simulink model "SdbRxSigProc"  (R2022b)
settings: Solver=FixedStepDiscrete  StartTime=0.0  StopTime=10.0  FixedStep=0.05  RelTol=1e-3
(part 3 of 4)

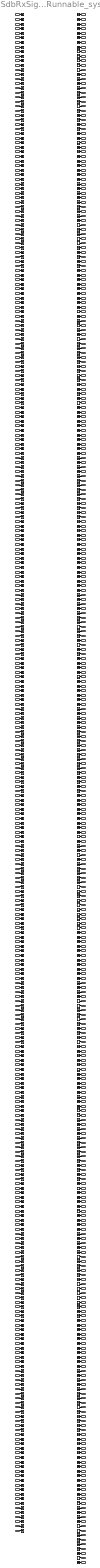
== system SdbRxSigProc/SdbRxSigProc_Runnable_sys ==
  ActvnOfWshrFrntSafe_ActvnOfWshrFrntSafe_read : Inport
  AirTFromHeatgEstimd_AirTFromHeatgEstimd_read : Inport  Port=2
  AmbTRaw_AmbTRaw_read : Inport  Port=3
  BltLockStAtDrvr_BltLockStAtDrvr_read : Inport  Port=4
  BltLockStAtPass_BltLockStAtPass_read : Inport  Port=5
  BltLockStAtRowSecLe_BltLockStAtRowSecLe_read : Inport  Port=6
  BltLockStAtRowSecMid_BltLockStAtRowSecMid_read : Inport  Port=7
  BltLockStAtRowSecRi_BltLockStAtRowSecRi_read : Inport  Port=8
  BltLockStAtRowThrdLe_BltLockStAtRowThrdLe_read : Inport  Port=9
  BltLockStAtRowThrdRi_BltLockStAtRowThrdRi_read : Inport  Port=10
  BookTrvlClimaActvReq_BookTrvlClimaActvReq_read : Inport  Port=11
  CamSts_CamSts_read : Inport  Port=12
  CarTiGlb_CarTiGlb_read : Inport  Port=13
  ClimaCfgForSnsrHumPrsnt1_ClimaCfgForSnsrHumPrsnt1_read : Inport  Port=14
  ClimaCmd_ClimaCmd_read : Inport  Port=15
  ClimaPwrAllwd_ClimaPwrAllwd_read : Inport  Port=16
  CmpmtInAirPmAQI_CmpmtInAirPmAQI_read : Inport  Port=17
  CmpmtInAirPmDnsty_CmpmtInAirPmDnsty_read : Inport  Port=18
  CmptmtCoolgSts_CmptmtCoolgSts_read : Inport  Port=19
  CmptmtFrntWindDewT_CmptmtFrntWindDewT_read : Inport  Port=20
  CmptmtFrntWindT_CmptmtFrntWindT_read : Inport  Port=21
  CmptmtRelHum_CmptmtRelHum_read : Inport  Port=22
  CoolgLoadGroup_CoolgLoadGroup_read : Inport  Port=23
  DefrstSts_DefrstSts_read : Inport  Port=24
  DoorDrvrSts_DoorDrvrSts_read : Inport  Port=25
  DoorLeReSts_DoorLeReSts_read : Inport  Port=26
  DoorPassSts_DoorPassSts_read : Inport  Port=27
  DoorRiReSts_DoorRiReSts_read : Inport  Port=28
  DrvModReq_DrvModReq_read : Inport  Port=29
  DrvrAirCdnrSetg_DrvrAirCdnrSetg_read : Inport  Port=30
  DrvrSeatHeatgActlPwrCns_DrvrSeatHeatgActlPwrCns_read : Inport  Port=31
  DrvrSeatHeatgDesPwrCns_DrvrSeatHeatgDesPwrCns_read : Inport  Port=32
  DrvrSeatVentnActlPwrCns_DrvrSeatVentnActlPwrCns_read : Inport  Port=33
  DrvrSeatVentnDesPwrCns_DrvrSeatVentnDesPwrCns_read : Inport  Port=34
  EgyCnsAllwdForClima_EgyCnsAllwdForClima_read : Inport  Port=35
  EngT_EngT_read : Inport  Port=36
  HMIClimaEgySaveReq_HMIClimaEgySaveReq_read : Inport  Port=37
  HpcDeiceReq_HpcDeiceReq_read : Inport  Port=38
  HvacFanSts2HvacFanBattI2_HvacFanSts2HvacFanBattI2_read : Inport  Port=39
  HvacFanSts2HvacFanMotU2_HvacFanSts2HvacFanMotU2_read : Inport  Port=40
  HvacFanSts3HvacFanBattI_HvacFanSts3HvacFanBattI_read : Inport  Port=41
  HvacFanSts3HvacFanSpdFd_HvacFanSts3HvacFanSpdFd_read : Inport  Port=42
  HvPwrEgyPrio_HvPwrEgyPrio_read : Inport  Port=43
  IHUAPSts_IHUAPSts_read : Inport  Port=44
  IncomingAirQlyCtrlFromHmi_IncomingAirQlyCtrlFromHmi_read : Inport  Port=45
  IncomingAirQlyPopUpReq_IncomingAirQlyPopUpReq_read : Inport  Port=46
  InPm25FanErr_InPm25FanErr_read : Inport  Port=47
  InPm25SnsrElecErr_InPm25SnsrElecErr_read : Inport  Port=48
  InPm25SnsrSts_InPm25SnsrSts_read : Inport  Port=49
  InteCleanUnpleSmell_InteCleanUnpleSmell_read : Inport  Port=50
  IsgMotT_IsgMotT_read : Inport  Port=51
  LeSolarData_LeSolarData_read : Inport  Port=52
  LockgCenSts_LockgCenSts_read : Inport  Port=53
  OnBdChrgrHndlSts1_OnBdChrgrHndlSts1_read : Inport  Port=54
  PassSeatHeatgActlPwrCns_PassSeatHeatgActlPwrCns_read : Inport  Port=55
  PassSeatHeatgDesPwrCns_PassSeatHeatgDesPwrCns_read : Inport  Port=56
  PassSeatVentnActlPwrCns_PassSeatVentnActlPwrCns_read : Inport  Port=57
  PassSeatVentnDesPwrCns_PassSeatVentnDesPwrCns_read : Inport  Port=58
  PostDrvgClimaReq_PostDrvgClimaReq_read : Inport  Port=59
  RainfallAmnt_RainfallAmnt_read : Inport  Port=60
  RelHumSnsrQf_RelHumSnsrQf_read : Inport  Port=61
  RemStrtHvCtrlReq_RemStrtHvCtrlReq_read : Inport  Port=62
  RiSolarData_RiSolarData_read : Inport  Port=63
  SceneModSeld_SceneModSeld_read : Inport  Port=64
  SeatHeatDurgClimaEnadFromTelm_SeatHeatDurgClimaEnadFromTelm_read : Inport  Port=65
  SecRowSeatHeatgActlPwrCnsLe_SecRowSeatHeatgActlPwrCnsLe_read : Inport  Port=66
  SecRowSeatHeatgActlPwrCnsRi_SecRowSeatHeatgActlPwrCnsRi_read : Inport  Port=67
  SecRowSeatHeatgDesPwrCnsLe_SecRowSeatHeatgDesPwrCnsLe_read : Inport  Port=68
  SecRowSeatHeatgDesPwrCnsRi_SecRowSeatHeatgDesPwrCnsRi_read : Inport  Port=69
  SecRowSeatVentnActlPwrCnsLe_SecRowSeatVentnActlPwrCnsLe_read : Inport  Port=70
  SecRowSeatVentnActlPwrCnsRi_SecRowSeatVentnActlPwrCnsRi_read : Inport  Port=71
  SecRowSeatVentnDesPwrCnsLe_SecRowSeatVentnDesPwrCnsLe_read : Inport  Port=72
  SecRowSeatVentnDesPwrCnsRi_SecRowSeatVentnDesPwrCnsRi_read : Inport  Port=73
  SolarSnsrVluQf_SolarSnsrVluQf_read : Inport  Port=74
  SteerWhlHeatgDesPwr_SteerWhlHeatgDesPwr_read : Inport  Port=75
  SteerWhlHeatgPwrAct_SteerWhlHeatgPwrAct_read : Inport  Port=76
  StrtRemSts1WdStrtRemSts_StrtRemSts1WdStrtRemSts_read : Inport  Port=77
  SunCurtPosnSts_SunCurtPosnSts_read : Inport  Port=78
  SunRoofPosnSts_SunRoofPosnSts_read : Inport  Port=79
  TelmPM25Req_TelmPM25Req_read : Inport  Port=80
  TrSts_TrSts_read : Inport  Port=81
  VehBattU_VehBattU_read : Inport  Port=82
  VehSpdLgt_VehSpdLgt_read : Inport  Port=83
  VehTiAndData_VehTiAndData_read : Inport  Port=84
  WadeProtnSts_WadeProtnSts_read : Inport  Port=85
  WinGlbCmd_WinGlbCmd_read : Inport  Port=86
  WinPosnStsAtDrvr_WinPosnStsAtDrvr_read : Inport  Port=87
  WinPosnStsAtPass_WinPosnStsAtPass_read : Inport  Port=88
  WinPosnStsAtReLe_WinPosnStsAtReLe_read : Inport  Port=89
  WinPosnStsAtReRi_WinPosnStsAtReRi_read : Inport  Port=90
  WipgInfo_WipgInfo_read : Inport  Port=91
  VehModMngtGlbSafe1_VehModMngtGlbSafe1_read : Inport  Port=92
  VehModMngtGlbSafe1_VehModMngtGlbSafe1_IsUpdated_read : Inport  Port=93
  AACAirClngErrSts_AACAirClngErrSts_read : Inport  Port=94
  AACAirClngSts_AACAirClngSts_read : Inport  Port=95
  AFUFragraCh1AvlTiPrec_AFUFragraCh1AvlTiPrec_read : Inport  Port=96
  AFUFragraCh1AvlTiPrec_AFUFragraCh1AvlTiPrec_IsUpdated_read : Inport  Port=97
  AFUFragraCh2AvlTiPrec_AFUFragraCh2AvlTiPrec_read : Inport  Port=98
  AFUFragraCh2AvlTiPrec_AFUFragraCh2AvlTiPrec_IsUpdated_read : Inport  Port=99
  AFUFragraCh3AvlTiPrec_AFUFragraCh3AvlTiPrec_read : Inport  Port=100
  AFUFragraCh3AvlTiPrec_AFUFragraCh3AvlTiPrec_IsUpdated_read : Inport  Port=101
  AFUFragraCh4AvlTiPrec_AFUFragraCh4AvlTiPrec_read : Inport  Port=102
  AFUFragraCh4AvlTiPrec_AFUFragraCh4AvlTiPrec_IsUpdated_read : Inport  Port=103
  AFUFragraCh5AvlTiPrec_AFUFragraCh5AvlTiPrec_read : Inport  Port=104
  AFUFragraCh5AvlTiPrec_AFUFragraCh5AvlTiPrec_IsUpdated_read : Inport  Port=105
  AirFragCh1OvrdDy_AirFragCh1OvrdDy_read : Inport  Port=106
  AirFragCh2OvrdDy_AirFragCh2OvrdDy_read : Inport  Port=107
  AirFragCh3OvrdDy_AirFragCh3OvrdDy_read : Inport  Port=108
  AirFragCh4OvrdDy_AirFragCh4OvrdDy_read : Inport  Port=109
  AirFragCh5OvrdDy_AirFragCh5OvrdDy_read : Inport  Port=110
  AirPtcEnad_AirPtcEnad_read : Inport  Port=111
  AirPtcHVPwrActl_AirPtcHVPwrActl_read : Inport  Port=112
  AirPtcHVPwrActlFristLe_AirPtcHVPwrActlFristLe_read : Inport  Port=113
  AirPtcHVPwrActlFristRi_AirPtcHVPwrActlFristRi_read : Inport  Port=114
  AirPtcHVPwrActlSecLe_AirPtcHVPwrActlSecLe_read : Inport  Port=115
  AirPtcModeReq_AirPtcModeReq_read : Inport  Port=116
  AirSrcStsFb_AirSrcStsFb_read : Inport  Port=117
  AltitudeInfrom_AltitudeInfrom_read : Inport  Port=118
  AMBSts_AMBSts_read : Inport  Port=119
  BackflushVlvErr_BackflushVlvErr_read : Inport  Port=120
  BusCurrentFb_BusCurrentFb_read : Inport  Port=121
  ChgFilElmWarn_ChgFilElmWarn_read : Inport  Port=122
  ClimaCfgForDrvrSeatVentWthSmf2_ClimaCfgForDrvrSeatVentWthSmf2_read : Inport  Port=123
  ClimaCfgForPassSeatVentWthSmf2_ClimaCfgForPassSeatVentWthSmf2_read : Inport  Port=124
  CmptmtOxygenConcQF_CmptmtOxygenConcQF_read : Inport  Port=125
  CmptmtOxygenConcVlu_CmptmtOxygenConcVlu_read : Inport  Port=126
  CoolgFanErr_CoolgFanErr_read : Inport  Port=127
  CourseDirFromNav_CourseDirFromNav_read : Inport  Port=128
  CurrentCongestionInformation_CurrentCongestionInformation_read : Inport  Port=129
  CurrentTunnelInformation_CurrentTunnelInformation_read : Inport  Port=130
  DcDcActvd_DcDcActvd_read : Inport  Port=131
  DcDcPwrLim_DcDcPwrLim_read : Inport  Port=132
  DHUResdSig10_DHUResdSig10_read : Inport  Port=133
  DHUResdSig11_DHUResdSig11_read : Inport  Port=134
  DHUResdSig12_DHUResdSig12_read : Inport  Port=135
  DHUResdSig13_DHUResdSig13_read : Inport  Port=136
  DHUResdSig3_DHUResdSig3_read : Inport  Port=137
  DHUResdSig4_DHUResdSig4_read : Inport  Port=138
  DHUResdSig5_DHUResdSig5_read : Inport  Port=139
  DHUResdSig6_DHUResdSig6_read : Inport  Port=140
  DHUResdSig7_DHUResdSig7_read : Inport  Port=141
  DHUResdSig8_DHUResdSig8_read : Inport  Port=142
  DHUResdSig9_DHUResdSig9_read : Inport  Port=143
  DispHvBattLvlOfChrg_DispHvBattLvlOfChrg_read : Inport  Port=144
  DropTopPosnSts_DropTopPosnSts_read : Inport  Port=145
  DrvModReqType1ForCompile_DrvModReqType1ForCompile_read : Inport  Port=146
  DrvrSeatPosPerc_DrvrSeatPosPerc_read : Inport  Port=147
  DrvrSeatPosPerc_DrvrSeatPosPerc_IsUpdated_read : Inport  Port=148
  DrvrSeatSts_DrvrSeatSts_read : Inport  Port=149
  DrvrSeatVentnActlPwrCns2_DrvrSeatVentnActlPwrCns2_read : Inport  Port=150
  DrvrSeatVentnDesPwrCns2_DrvrSeatVentnDesPwrCns2_read : Inport  Port=151
  DrvrSeatVentnLvlSts2_DrvrSeatVentnLvlSts2_read : Inport  Port=152
  EngSt1WdSts_EngSt1WdSts_read : Inport  Port=153
  EngSt1WdSts_EngSt1WdSts_IsUpdated_read : Inport  Port=154
  FctvSts_FctvSts_read : Inport  Port=155
  FloatDrvingActv_FloatDrvingActv_read : Inport  Port=156
  FrntAirPtcHVPwrActl_FrntAirPtcHVPwrActl_read : Inport  Port=157
  FrntAirPtcHVPwrActl_FrntAirPtcHVPwrActl_IsUpdated_read : Inport  Port=158
  HighTempCleanHmiReq_HighTempCleanHmiReq_read : Inport  Port=159
  HmiAC_HmiAC_read : Inport  Port=160
  HmiAC_HmiAC_IsUpdated_read : Inport  Port=161
  HMIAirCdnrAutDrySet_HMIAirCdnrAutDrySet_read : Inport  Port=162
  HMIAirCdnrAutDrySet_HMIAirCdnrAutDrySet_IsUpdated_read : Inport  Port=163
  HmiAirDistbnRow1_HmiAirDistbnRow1_read : Inport  Port=164
  HmiAirDistbnRow1_HmiAirDistbnRow1_IsUpdated_read : Inport  Port=165
  HmiAirDistbnRow1Le_HmiAirDistbnRow1Le_read : Inport  Port=166
  HmiAirDistbnRow1Ri_HmiAirDistbnRow1Ri_read : Inport  Port=167
  HmiAirDistbnRow2_HmiAirDistbnRow2_read : Inport  Port=168
  HmiAirDistbnRow2_HmiAirDistbnRow2_IsUpdated_read : Inport  Port=169
  HmiAutoRow1_HmiAutoRow1_read : Inport  Port=170
  HmiAutoRow1_HmiAutoRow1_IsUpdated_read : Inport  Port=171
  HmiAutoRow1Le_HmiAutoRow1Le_read : Inport  Port=172
  HmiAutoRow1Ri_HmiAutoRow1Ri_read : Inport  Port=173
  HmiAutoRow2_HmiAutoRow2_read : Inport  Port=174
  HmiAutoRow2_HmiAutoRow2_IsUpdated_read : Inport  Port=175
  HmiAutOxySet_HmiAutOxySet_read : Inport  Port=176
  HMIBluetoothCallToAdjAirVol_HMIBluetoothCallToAdjAirVol_read : Inport  Port=177
  HmiCarLockACRecircSet_HmiCarLockACRecircSet_read : Inport  Port=178
  HmiCbnOverHeatPrevnReq_HmiCbnOverHeatPrevnReq_read : Inport  Port=179
  HmiCbnOverHeatPrevnReq_HmiCbnOverHeatPrevnReq_IsUpdated_read : Inport  Port=180
  HmiClimaRow1LePwr_HmiClimaRow1LePwr_read : Inport  Port=181
  HmiClimaRow1Pwr_HmiClimaRow1Pwr_read : Inport  Port=182
  HmiClimaRow1RiPwr_HmiClimaRow1RiPwr_read : Inport  Port=183
  HmiClimaRow2Pwr_HmiClimaRow2Pwr_read : Inport  Port=184
  HmiClimaRow3Pwr_HmiClimaRow3Pwr_read : Inport  Port=185
  HMIClimaVentSet_HMIClimaVentSet_read : Inport  Port=186
  HmiCmptmtAirDistbnFrnt_HmiCmptmtAirDistbnFrnt_read : Inport  Port=187
  HmiDrvrHeadPosn_HmiDrvrHeadPosn_read : Inport  Port=188
  HmiEcoClimaReq_HmiEcoClimaReq_read : Inport  Port=189
  HmiEcoClimaReq_HmiEcoClimaReq_IsUpdated_read : Inport  Port=190
  HmiElecAirBodyVentLebtn_HmiElecAirBodyVentLebtn_read : Inport  Port=191
  HmiElecAirBodyVentRibtn_HmiElecAirBodyVentRibtn_read : Inport  Port=192
  HmiElecAirConsolePosn_HmiElecAirConsolePosn_read : Inport  Port=193
  HmiElecAirConsoleSwtLe_HmiElecAirConsoleSwtLe_read : Inport  Port=194
  HmiElecAirConsoleSwtRi_HmiElecAirConsoleSwtRi_read : Inport  Port=195
  HmiElecAirDirModeSecRowReq_HmiElecAirDirModeSecRowReq_read : Inport  Port=196
  HmiElecAirDirSwngSecLe_HmiElecAirDirSwngSecLe_read : Inport  Port=197
  HmiElecAirDirSwngSecRi_HmiElecAirDirSwngSecRi_read : Inport  Port=198
  HmiElecAirDrvrNatureWindReq_HmiElecAirDrvrNatureWindReq_read : Inport  Port=199
  HmiElecAirPassNatureWindReq_HmiElecAirPassNatureWindReq_read : Inport  Port=200
  HMIFACSwt_HMIFACSwt_read : Inport  Port=201
  HmiFanLvlRow1_HmiFanLvlRow1_read : Inport  Port=202
  HmiFanLvlRow1_HmiFanLvlRow1_IsUpdated_read : Inport  Port=203
  HmiFanLvlRow1Le_HmiFanLvlRow1Le_read : Inport  Port=204
  HmiFanLvlRow1Ri_HmiFanLvlRow1Ri_read : Inport  Port=205
  HmiFanLvlRow2_HmiFanLvlRow2_read : Inport  Port=206
  HmiFanLvlRow2_HmiFanLvlRow2_IsUpdated_read : Inport  Port=207
  HmiFragraCh_HmiFragraCh_read : Inport  Port=208
  HmiFragraCh_HmiFragraCh_IsUpdated_read : Inport  Port=209
  HmiFragraLvl_HmiFragraLvl_read : Inport  Port=210
  HmiFragraLvl_HmiFragraLvl_IsUpdated_read : Inport  Port=211
  HMIIonizerAirCdnr_HMIIonizerAirCdnr_read : Inport  Port=212
  HMIIonizerAirCdnr_HMIIonizerAirCdnr_IsUpdated_read : Inport  Port=213
  HMILBRACStrtSet_HMILBRACStrtSet_read : Inport  Port=214
  HmiMaxAC_HmiMaxAC_read : Inport  Port=215
  HmiMaxAC_HmiMaxAC_IsUpdated_read : Inport  Port=216
  HmiMaxDefrst_HmiMaxDefrst_read : Inport  Port=217
  HmiMaxDefrst_HmiMaxDefrst_IsUpdated_read : Inport  Port=218
  HmiOxyinCarSwt_HmiOxyinCarSwt_read : Inport  Port=219
  HmiParkVentSet_HmiParkVentSet_read : Inport  Port=220
  HmiParkVentTmrSet_HmiParkVentTmrSet_read : Inport  Port=221
  HmiPassHeadPosn_HmiPassHeadPosn_read : Inport  Port=222
  HmiPM25PopUpResp_HmiPM25PopUpResp_read : Inport  Port=223
  HmiPM25PopUpResp_HmiPM25PopUpResp_IsUpdated_read : Inport  Port=224
  HmiPM25PopUpSet_HmiPM25PopUpSet_read : Inport  Port=225
  HmiPM25PopUpSet_HmiPM25PopUpSet_IsUpdated_read : Inport  Port=226
  HmiSmrtWindFolwSet_HmiSmrtWindFolwSet_read : Inport  Port=227
  HMIThermoTankDoorOpen_HMIThermoTankDoorOpen_read : Inport  Port=228
  HMIThermoTankEcoSw_HMIThermoTankEcoSw_read : Inport  Port=229
  HMIThermoTankT_HMIThermoTankT_read : Inport  Port=230
  HmiThermoTSteDeoNewSwt_HmiThermoTSteDeoNewSwt_read : Inport  Port=231
  HmiThermoTSteDeoSwt_HmiThermoTSteDeoSwt_read : Inport  Port=232
  HmiTSyncn_HmiTSyncn_read : Inport  Port=233
  HmiTSyncn_HmiTSyncn_IsUpdated_read : Inport  Port=234
  HmiUVSterilSet_HmiUVSterilSet_read : Inport  Port=235
  HmiWinterLockCarPrevnIceSet_HmiWinterLockCarPrevnIceSet_read : Inport  Port=236
  HmiWinterLockCarPrevnIceSet_HmiWinterLockCarPrevnIceSet_IsUpdated_read : Inport  Port=237
  HoodSts_HoodSts_read : Inport  Port=238
  HvBattLimnIndcn_HvBattLimnIndcn_read : Inport  Port=239
  InflateAirSrcSwt_InflateAirSrcSwt_read : Inport  Port=240
  InpVoltFb_InpVoltFb_read : Inport  Port=241
  LiOprnMod_LiOprnMod_read : Inport  Port=242
  LonglifeBatteryReq_LonglifeBatteryReq_read : Inport  Port=243
  McuOvrTempErr_McuOvrTempErr_read : Inport  Port=244
  McuTempFb_McuTempFb_read : Inport  Port=245
  McuTempSnsrErr_McuTempSnsrErr_read : Inport  Port=246
  MoleculeVlv1_MoleculeVlv1_read : Inport  Port=247
  MoleculeVlv2_MoleculeVlv2_read : Inport  Port=248
  MosOvrTempErr_MosOvrTempErr_read : Inport  Port=249
  MosTempFb_MosTempFb_read : Inport  Port=250
  MosTempSnsrErr_MosTempSnsrErr_read : Inport  Port=251
  MotStsFrmOGM_MotStsFrmOGM_read : Inport  Port=252
  MotTempFb_MotTempFb_read : Inport  Port=253
  MotTempSnsrErr_MotTempSnsrErr_read : Inport  Port=254
  OGMCmprSpdFb_OGMCmprSpdFb_read : Inport  Port=255
  OGMFlowFb_OGMFlowFb_read : Inport  Port=256
  OGMHwVers_OGMHwVers_read : Inport  Port=257
  OGMIntErr_OGMIntErr_read : Inport  Port=258
  OGMOvrVoltRangErr_OGMOvrVoltRangErr_read : Inport  Port=259
  OGMRunTi_OGMRunTi_read : Inport  Port=260
  OGMSwVers_OGMSwVers_read : Inport  Port=261
  OGMTempErr_OGMTempErr_read : Inport  Port=262
  OGMUndVoltRangErr_OGMUndVoltRangErr_read : Inport  Port=263
  OxygenConcFbFrmOGM_OxygenConcFbFrmOGM_read : Inport  Port=264
  OxygenConcFlt_OxygenConcFlt_read : Inport  Port=265
  OxygenConcSnsrElecErr_OxygenConcSnsrElecErr_read : Inport  Port=266
  OxygenConcSnsrIntErr_OxygenConcSnsrIntErr_read : Inport  Port=267
  OxygenConcSnsrOvrVoltRangErr_OxygenConcSnsrOvrVoltRangErr_read : Inport  Port=268
  OxygenConcSnsrSts_OxygenConcSnsrSts_read : Inport  Port=269
  OxygenConcSnsrStsFrmOGM_OxygenConcSnsrStsFrmOGM_read : Inport  Port=270
  OxygenConcSnsrTempErr_OxygenConcSnsrTempErr_read : Inport  Port=271
  OxygenConcSnsrUndVoltRangErr_OxygenConcSnsrUndVoltRangErr_read : Inport  Port=272
  OxygenGenrErrInfo_OxygenGenrErrInfo_read : Inport  Port=273
  OxygenSplyAvlSts_OxygenSplyAvlSts_read : Inport  Port=274
  OxygenSplyErr_OxygenSplyErr_read : Inport  Port=275
  OxygenTubeBlk_OxygenTubeBlk_read : Inport  Port=276
  PassSeatPosPerc_PassSeatPosPerc_read : Inport  Port=277
  PassSeatPosPerc_PassSeatPosPerc_IsUpdated_read : Inport  Port=278
  PassSeatSts2_PassSeatSts2_read : Inport  Port=279
  PassSeatVentnActlPwrCns2_PassSeatVentnActlPwrCns2_read : Inport  Port=280
  PassSeatVentnDesPwrCns2_PassSeatVentnDesPwrCns2_read : Inport  Port=281
  PassSeatVentnLvlSts2_PassSeatVentnLvlSts2_read : Inport  Port=282
  PhaseCurrentFb_PhaseCurrentFb_read : Inport  Port=283
  PosnBrief_PosnBrief_read : Inport  Port=284
  PressureFb_PressureFb_read : Inport  Port=285
  PressureFlt_PressureFlt_read : Inport  Port=286
  PressureSnsrErr_PressureSnsrErr_read : Inport  Port=287
  RefrLpSwSts_RefrLpSwSts_read : Inport  Port=288
  RefrtorVlvEna_RefrtorVlvEna_read : Inport  Port=289
  ResrvdSigForCCM2_ResrvdSigForCCM2_read : Inport  Port=290
  RoadIncln_RoadIncln_read : Inport  Port=291
  RuranACModeSet_RuranACModeSet_read : Inport  Port=292
  SeatBackAngleRowFirstDrvr_SeatBackAngleRowFirstDrvr_read : Inport  Port=293
  SeatBackAngleRowFirstDrvr_SeatBackAngleRowFirstDrvr_IsUpdated_read : Inport  Port=294
  SeatBackAngleRowFirstPass_SeatBackAngleRowFirstPass_read : Inport  Port=295
  SeatBackAngleRowFirstPass_SeatBackAngleRowFirstPass_IsUpdated_read : Inport  Port=296
  SeatOccptAtRowSecDrSide2_SeatOccptAtRowSecDrSide2_read : Inport  Port=297
  SeatOccptAtRowSecMid2_SeatOccptAtRowSecMid2_read : Inport  Port=298
  SeatOccptAtRowSecPasSide2_SeatOccptAtRowSecPasSide2_read : Inport  Port=299
  SeatOccptAtRowThrdLe2_SeatOccptAtRowThrdLe2_read : Inport  Port=300
  SeatOccptAtRowThrdRi2_SeatOccptAtRowThrdRi2_read : Inport  Port=301
  SportModeThmgtRequest_SportModeThmgtRequest_read : Inport  Port=302
  TCSCooltFlow_TCSCooltFlow_read : Inport  Port=303
  TCSCooltT_TCSCooltT_read : Inport  Port=304
  TcsDoorEleBailReq_TcsDoorEleBailReq_read : Inport  Port=305
  TcsFanReq_TcsFanReq_read : Inport  Port=306
  TcsModSwtReq_TcsModSwtReq_read : Inport  Port=307
  TcsOvrheatdProtnSts_TcsOvrheatdProtnSts_read : Inport  Port=308
  TcsSemiconReq_TcsSemiconReq_read : Inport  Port=309
  TcsTmrOverReq_TcsTmrOverReq_read : Inport  Port=310
  TcsTNowCtrlReq_TcsTNowCtrlReq_read : Inport  Port=311
  TcsTopDoorEleBailReq_TcsTopDoorEleBailReq_read : Inport  Port=312
  TelmOxygenValReq_TelmOxygenValReq_read : Inport  Port=313
  TelmThermoTankECOSet_TelmThermoTankECOSet_read : Inport  Port=314
  TelmThermoTankT_TelmThermoTankT_read : Inport  Port=315
  ThermHvacRecircReq_ThermHvacRecircReq_read : Inport  Port=316
  ThermHvacRecircReqEna_ThermHvacRecircReqEna_read : Inport  Port=317
  ThrdRowSeatHeatgActlPwrCnsLe_ThrdRowSeatHeatgActlPwrCnsLe_read : Inport  Port=318
  ThrdRowSeatHeatgActlPwrCnsRi_ThrdRowSeatHeatgActlPwrCnsRi_read : Inport  Port=319
  ThrdRowSeatHeatgDesPwrCnsLe_ThrdRowSeatHeatgDesPwrCnsLe_read : Inport  Port=320
  ThrdRowSeatHeatgDesPwrCnsRi_ThrdRowSeatHeatgDesPwrCnsRi_read : Inport  Port=321
  ThrdRowSeatVentnActlPwrCnsLe_ThrdRowSeatVentnActlPwrCnsLe_read : Inport  Port=322
  ThrdRowSeatVentnActlPwrCnsRi_ThrdRowSeatVentnActlPwrCnsRi_read : Inport  Port=323
  ThrdRowSeatVentnDesPwrCnsLe_ThrdRowSeatVentnDesPwrCnsLe_read : Inport  Port=324
  ThrdRowSeatVentnDesPwrCnsRi_ThrdRowSeatVentnDesPwrCnsRi_read : Inport  Port=325
  UseEvapReqTMinNew_UseEvapReqTMinNew_read : Inport  Port=326
  VlvVoltFlt_VlvVoltFlt_read : Inport  Port=327
  WarnLvlMsg_WarnLvlMsg_read : Inport  Port=328
  WarnLvlMsg_WarnLvlMsg_IsUpdated_read : Inport  Port=329
  WasRespLevel_WasRespLevel_read : Inport  Port=330
  WasRespLevel_WasRespLevel_IsUpdated_read : Inport  Port=331
  WaterDepthInformation_WaterDepthInformation_read : Inport  Port=332
  WeatherQualityAQI_WeatherQualityAQI_read : Inport  Port=333
  function : TriggerPort  TriggerType=function-call  VariantControl=(inherit)  SampleTimeType=periodic  SampleTime=-1
  Ground_{1..8,14..20,22..28,30..46,48..49,52..65,67..68,70..71,73..79,81..85,87..134,136..138,140..145,147..180,182..184,186..191,194,202..216,219..220,222..225,227..231,233..240,243..257,259..262,264..272,274..278,284..305,307..325,328..331,334..338,340} (290 alike) : Ground
  Ground_10 : Subsystem
  Ground_11 : Subsystem
  Ground_12 : Subsystem
  Ground_13 : Subsystem
  Ground_135 : Subsystem
  Ground_139 : Subsystem
  Ground_146 : Subsystem
  Ground_181 : Subsystem
  Ground_185 : Subsystem
  Ground_192 : Subsystem
  Ground_193 : Subsystem
  Ground_195 : Subsystem
  Ground_196 : Subsystem
  Ground_197 : Subsystem
  Ground_198 : Subsystem
  Ground_199 : Subsystem
  Ground_200 : Subsystem
  Ground_201 : Subsystem
  Ground_21 : Subsystem
  Ground_217 : Subsystem
  Ground_218 : Subsystem
  Ground_221 : Subsystem
  Ground_226 : Subsystem
  Ground_232 : Subsystem
  Ground_241 : Subsystem
  Ground_242 : Subsystem
  Ground_258 : Subsystem
  Ground_263 : Subsystem
  Ground_273 : Subsystem
  Ground_279 : Subsystem
  Ground_280 : Subsystem
  Ground_281 : Subsystem
  Ground_282 : Subsystem
  Ground_283 : Subsystem
  Ground_29 : Subsystem
  Ground_306 : Subsystem
  Ground_326 : Subsystem
  Ground_327 : Subsystem
  Ground_332 : Subsystem
  Ground_333 : Subsystem
  Ground_339 : Subsystem
  Ground_47 : Subsystem
  Ground_50 : Subsystem
  Ground_51 : Subsystem
  Ground_66 : Subsystem
  Ground_69 : Subsystem
  Ground_72 : Subsystem
  Ground_80 : Subsystem
  Ground_86 : Subsystem
  Ground_9 : Subsystem
  Terminator_{1..333} (333 alike) : Terminator
  AACAirClngErrStsNgcc_AACAirClngErrStsNgcc_write : Outport
      settings: EnsureOutportIsVirtual=on
  AACAirClngStsNgcc_AACAirClngStsNgcc_write : Outport  Port=2
      settings: EnsureOutportIsVirtual=on
  AFUFragraCh1AvlTiPrecNgcc_AFUFragraCh1AvlTiPrecNgcc_write : Outport  Port=3
      settings: EnsureOutportIsVirtual=on
  AFUFragraCh2AvlTiPrecNgcc_AFUFragraCh2AvlTiPrecNgcc_write : Outport  Port=4
      settings: EnsureOutportIsVirtual=on
  AFUFragraCh3AvlTiPrecNgcc_AFUFragraCh3AvlTiPrecNgcc_write : Outport  Port=5
      settings: EnsureOutportIsVirtual=on
  AFUFragraCh4AvlTiPrecNgcc_AFUFragraCh4AvlTiPrecNgcc_write : Outport  Port=6
      settings: EnsureOutportIsVirtual=on
  AFUFragraCh5AvlTiPrecNgcc_AFUFragraCh5AvlTiPrecNgcc_write : Outport  Port=7
      settings: EnsureOutportIsVirtual=on
  AirClngReq_AirClngReq_write : Outport  Port=8
      settings: EnsureOutportIsVirtual=on
  AirFragCh1OvrdDyNgcc_AirFragCh1OvrdDyNgcc_write : Outport  Port=9
      settings: EnsureOutportIsVirtual=on
  AirFragCh2OvrdDyNgcc_AirFragCh2OvrdDyNgcc_write : Outport  Port=10
      settings: EnsureOutportIsVirtual=on
  AirFragCh3OvrdDyNgcc_AirFragCh3OvrdDyNgcc_write : Outport  Port=11
      settings: EnsureOutportIsVirtual=on
  AirFragCh4OvrdDyNgcc_AirFragCh4OvrdDyNgcc_write : Outport  Port=12
      settings: EnsureOutportIsVirtual=on
  AirFragCh5OvrdDyNgcc_AirFragCh5OvrdDyNgcc_write : Outport  Port=13
      settings: EnsureOutportIsVirtual=on
  AirPtcEnadNgcc_AirPtcEnadNgcc_write : Outport  Port=14
      settings: EnsureOutportIsVirtual=on
  AirPtcHVPwrActlFirstLeNgcc_AirPtcHVPwrActlFirstLeNgcc_write : Outport  Port=15
      settings: EnsureOutportIsVirtual=on
  AirPtcHVPwrActlFirstRiNgcc_AirPtcHVPwrActlFirstRiNgcc_write : Outport  Port=16
      settings: EnsureOutportIsVirtual=on
  AirPtcHVPwrActlSecLeNgcc_AirPtcHVPwrActlSecLeNgcc_write : Outport  Port=17
      settings: EnsureOutportIsVirtual=on
  AirPtcModeReqNgcc_AirPtcModeReqNgcc_write : Outport  Port=18
      settings: EnsureOutportIsVirtual=on
  AirSrcStsFbNgcc_AirSrcStsFbNgcc_write : Outport  Port=19
      settings: EnsureOutportIsVirtual=on
  AltitudeInfromNgcc_AltitudeInfromNgcc_write : Outport  Port=20
      settings: EnsureOutportIsVirtual=on
  AMBStsNgcc_AMBStsNgcc_write : Outport  Port=21
      settings: EnsureOutportIsVirtual=on
  BackflushVlvErrNgcc_BackflushVlvErrNgcc_write : Outport  Port=22
      settings: EnsureOutportIsVirtual=on
  BookTrvlClimaActvReq_Ngcc_BookTrvlClimaActvReq_write : Outport  Port=23
      settings: EnsureOutportIsVirtual=on
  BusCurrentFbNgcc_BusCurrentFbNgcc_write : Outport  Port=24
      settings: EnsureOutportIsVirtual=on
  ChgFilElmWarnNgcc_ChgFilElmWarnNgcc_write : Outport  Port=25
      settings: EnsureOutportIsVirtual=on
  CmptmtOxygenConcQFNgcc_CmptmtOxygenConcQFNgcc_write : Outport  Port=26
      settings: EnsureOutportIsVirtual=on
  CmptmtOxygenConcVluNgcc_CmptmtOxygenConcVluNgcc_write : Outport  Port=27
      settings: EnsureOutportIsVirtual=on
  CoolgFanErrNgcc_CoolgFanErrNgcc_write : Outport  Port=28
      settings: EnsureOutportIsVirtual=on
  CourseDirFromNavNgcc_CourseDirFromNavNgcc_write : Outport  Port=29
      settings: EnsureOutportIsVirtual=on
  CurrentCongestionInformationNgcc_CurrentCongestionInformationNgcc_write : Outport  Port=30
      settings: EnsureOutportIsVirtual=on
  CurrentTunnelInformationNgcc_CurrentTunnelInformationNgcc_write : Outport  Port=31
      settings: EnsureOutportIsVirtual=on
  DcDcActvdNgcc_DcDcActvdNgcc_write : Outport  Port=32
      settings: EnsureOutportIsVirtual=on
  DcDcPwrLimNgcc_DcDcPwrLimNgcc_write : Outport  Port=33
      settings: EnsureOutportIsVirtual=on
  DHUResdSig10Ngcc_DHUResdSig10Ngcc_write : Outport  Port=34
      settings: EnsureOutportIsVirtual=on
  DHUResdSig11Ngcc_DHUResdSig11Ngcc_write : Outport  Port=35
      settings: EnsureOutportIsVirtual=on
  DHUResdSig12Ngcc_DHUResdSig12Ngcc_write : Outport  Port=36
      settings: EnsureOutportIsVirtual=on
  DHUResdSig13Ngcc_DHUResdSig13Ngcc_write : Outport  Port=37
      settings: EnsureOutportIsVirtual=on
  DHUResdSig3Ngcc_DHUResdSig3Ngcc_write : Outport  Port=38
      settings: EnsureOutportIsVirtual=on
  DHUResdSig4Ngcc_DHUResdSig4Ngcc_write : Outport  Port=39
      settings: EnsureOutportIsVirtual=on
  DHUResdSig5Ngcc_DHUResdSig5Ngcc_write : Outport  Port=40
      settings: EnsureOutportIsVirtual=on
  DHUResdSig6Ngcc_DHUResdSig6Ngcc_write : Outport  Port=41
      settings: EnsureOutportIsVirtual=on
  DHUResdSig7Ngcc_DHUResdSig7Ngcc_write : Outport  Port=42
      settings: EnsureOutportIsVirtual=on
  DHUResdSig8Ngcc_DHUResdSig8Ngcc_write : Outport  Port=43
      settings: EnsureOutportIsVirtual=on
  DHUResdSig9Ngcc_DHUResdSig9Ngcc_write : Outport  Port=44
      settings: EnsureOutportIsVirtual=on
  DispHvBattLvlOfChrgNgcc_DispHvBattLvlOfChrgNgcc_write : Outport  Port=45
      settings: EnsureOutportIsVirtual=on
  DropTopPosnStsNgcc_DropTopPosnStsNgcc_write : Outport  Port=46
      settings: EnsureOutportIsVirtual=on
  DrvrSeatPosPercNgcc_DrvrSeatPosPercNgcc_write : Outport  Port=47
      settings: EnsureOutportIsVirtual=on
  DrvrSeatSts_Ngcc_DrvrSeatSts_write : Outport  Port=48
      settings: EnsureOutportIsVirtual=on
  DrvrSeatVentnLvlSts2Ngcc_DrvrSeatVentnLvlSts2Ngcc_write : Outport  Port=49
      settings: EnsureOutportIsVirtual=on
  FctvStsNgcc_FctvStsNgcc_write : Outport  Port=50
      settings: EnsureOutportIsVirtual=on
  FloatDrvingActvNgcc_FloatDrvingActvNgcc_write : Outport  Port=51
      settings: EnsureOutportIsVirtual=on
  HighTempCleanHmiReqNgcc_HighTempCleanHmiReqNgcc_write : Outport  Port=52
      settings: EnsureOutportIsVirtual=on
  HMIAirCdnrAutDrySetNgcc_HMIAirCdnrAutDrySetNgcc_write : Outport  Port=53
      settings: EnsureOutportIsVirtual=on
  HmiAirDistbnRow1LeNgcc_HmiAirDistbnRow1LeNgcc_write : Outport  Port=54
      settings: EnsureOutportIsVirtual=on
  HmiAirDistbnRow1RiNgcc_HmiAirDistbnRow1RiNgcc_write : Outport  Port=55
      settings: EnsureOutportIsVirtual=on
  HmiAutoRow1LeNgcc_HmiAutoRow1LeNgcc_write : Outport  Port=56
      settings: EnsureOutportIsVirtual=on
  HmiAutoRow1RiNgcc_HmiAutoRow1RiNgcc_write : Outport  Port=57
      settings: EnsureOutportIsVirtual=on
  HmiAutOxySetNgcc_HmiAutOxySetNgcc_write : Outport  Port=58
      settings: EnsureOutportIsVirtual=on
  HmiCarLockACRecircSetNgcc_HmiCarLockACRecircSetNgcc_write : Outport  Port=59
      settings: EnsureOutportIsVirtual=on
  HmiClimaRow1LePwrNgcc_HmiClimaRow1LePwrNgcc_write : Outport  Port=60
      settings: EnsureOutportIsVirtual=on
  HmiClimaRow1PwrNgcc_HmiClimaRow1PwrNgcc_write : Outport  Port=61
      settings: EnsureOutportIsVirtual=on
  HmiClimaRow1RiPwrNgcc_HmiClimaRow1RiPwrNgcc_write : Outport  Port=62
      settings: EnsureOutportIsVirtual=on
  HmiClimaRow2PwrNgcc_HmiClimaRow2PwrNgcc_write : Outport  Port=63
      settings: EnsureOutportIsVirtual=on
  HmiClimaRow3PwrNgcc_HmiClimaRow3PwrNgcc_write : Outport  Port=64
      settings: EnsureOutportIsVirtual=on
  HMIClimaVentSetNgcc_HMIClimaVentSetNgcc_write : Outport  Port=65
      settings: EnsureOutportIsVirtual=on
  HmiDrvrHeadPosnNgcc_HmiDrvrHeadPosnNgcc_write : Outport  Port=66
      settings: EnsureOutportIsVirtual=on
  HmiElecAirBodyVentLebtnNgcc_HmiElecAirBodyVentLebtnNgcc_write : Outport  Port=67
      settings: EnsureOutportIsVirtual=on
  HmiElecAirBodyVentRibtnNgcc_HmiElecAirBodyVentRibtnNgcc_write : Outport  Port=68
      settings: EnsureOutportIsVirtual=on
  HmiElecAirConsolePosnNgcc_HmiElecAirConsolePosnNgcc_write : Outport  Port=69
      settings: EnsureOutportIsVirtual=on
  HmiElecAirConsoleSwtLeNgcc_HmiElecAirConsoleSwtLeNgcc_write : Outport  Port=70
      settings: EnsureOutportIsVirtual=on
  HmiElecAirConsoleSwtRiNgcc_HmiElecAirConsoleSwtRiNgcc_write : Outport  Port=71
      settings: EnsureOutportIsVirtual=on
  HmiElecAirDirModeSecRowReqNgcc_HmiElecAirDirModeSecRowReqNgcc_write : Outport  Port=72
      settings: EnsureOutportIsVirtual=on
  HmiElecAirDirSwngSecLeNgcc_HmiElecAirDirSwngSecLeNgcc_write : Outport  Port=73
      settings: EnsureOutportIsVirtual=on
  HmiElecAirDirSwngSecRiNgcc_HmiElecAirDirSwngSecRiNgcc_write : Outport  Port=74
      settings: EnsureOutportIsVirtual=on
  HmiElecAirDrvrNatureWindReqNgcc_HmiElecAirDrvrNatureWindReqNgcc_write : Outport  Port=75
      settings: EnsureOutportIsVirtual=on
  HmiElecAirPassNatureWindReqNgcc_HmiElecAirPassNatureWindReqNgcc_write : Outport  Port=76
      settings: EnsureOutportIsVirtual=on
  HMIFACSwtNgcc_HMIFACSwtNgcc_write : Outport  Port=77
      settings: EnsureOutportIsVirtual=on
  HmiFanLvlRow1LeNgcc_HmiFanLvlRow1LeNgcc_write : Outport  Port=78
      settings: EnsureOutportIsVirtual=on
  HmiFanLvlRow1RiNgcc_HmiFanLvlRow1RiNgcc_write : Outport  Port=79
      settings: EnsureOutportIsVirtual=on
  HmiFragraChRatReq_HmiFragraChRatReq_write : Outport  Port=80
      settings: EnsureOutportIsVirtual=on
  HmiFragraLvlReq_HmiFragraLvlReq_write : Outport  Port=81
      settings: EnsureOutportIsVirtual=on
  HMILBRACStrtSetNgcc_HMILBRACStrtSetNgcc_write : Outport  Port=82
      settings: EnsureOutportIsVirtual=on
  HmiOxyinCarSwtNgcc_HmiOxyinCarSwtNgcc_write : Outport  Port=83
      settings: EnsureOutportIsVirtual=on
  HmiParkVentSetNgcc_HmiParkVentSetNgcc_write : Outport  Port=84
      settings: EnsureOutportIsVirtual=on
  HmiParkVentTmrSetNgcc_HmiParkVentTmrSetNgcc_write : Outport  Port=85
      settings: EnsureOutportIsVirtual=on
  HmiPassHeadPosnNgcc_HmiPassHeadPosnNgcc_write : Outport  Port=86
      settings: EnsureOutportIsVirtual=on
  HmiPopUpResp_HmiPopUpResp_write : Outport  Port=87
      settings: EnsureOutportIsVirtual=on
  HmiSmrtWindFolwSetNgcc_HmiSmrtWindFolwSetNgcc_write : Outport  Port=88
      settings: EnsureOutportIsVirtual=on
  HMIThermoTankDoorOpenNgcc_HMIThermoTankDoorOpenNgcc_write : Outport  Port=89
      settings: EnsureOutportIsVirtual=on
  HMIThermoTankEcoSwNgcc_HMIThermoTankEcoSwNgcc_write : Outport  Port=90
      settings: EnsureOutportIsVirtual=on
  HMIThermoTankTNgcc_HMIThermoTankTNgcc_write : Outport  Port=91
      settings: EnsureOutportIsVirtual=on
  HmiThermoTSteDeoNewSwtNgcc_HmiThermoTSteDeoNewSwtNgcc_write : Outport  Port=92
      settings: EnsureOutportIsVirtual=on
  HmiThermoTSteDeoSwtNgcc_HmiThermoTSteDeoSwtNgcc_write : Outport  Port=93
      settings: EnsureOutportIsVirtual=on
  HmiUVSterilSetNgcc_HmiUVSterilSetNgcc_write : Outport  Port=94
      settings: EnsureOutportIsVirtual=on
  HmiWinterLockCarPrevnIceSetNgcc_HmiWinterLockCarPrevnIceSetNgcc_write : Outport  Port=95
      settings: EnsureOutportIsVirtual=on
  HoodStsNgcc_HoodStsNgcc_write : Outport  Port=96
      settings: EnsureOutportIsVirtual=on
  HvBattLimnIndcnNgcc_HvBattLimnIndcnNgcc_write : Outport  Port=97
      settings: EnsureOutportIsVirtual=on
  InflateAirSrcSwtNgcc_InflateAirSrcSwtNgcc_write : Outport  Port=98
      settings: EnsureOutportIsVirtual=on
  InpVoltFbNgcc_InpVoltFbNgcc_write : Outport  Port=99
      settings: EnsureOutportIsVirtual=on
  LiOprnModNgcc_LiOprnModNgcc_write : Outport  Port=100
      settings: EnsureOutportIsVirtual=on
  LonglifeBatteryReqNgcc_LonglifeBatteryReqNgcc_write : Outport  Port=101
      settings: EnsureOutportIsVirtual=on
  McuOvrTempErrNgcc_McuOvrTempErrNgcc_write : Outport  Port=102
      settings: EnsureOutportIsVirtual=on
  McuTempFbNgcc_McuTempFbNgcc_write : Outport  Port=103
      settings: EnsureOutportIsVirtual=on
  McuTempSnsrErrNgcc_McuTempSnsrErrNgcc_write : Outport  Port=104
      settings: EnsureOutportIsVirtual=on
  MoleculeVlv1Ngcc_MoleculeVlv1Ngcc_write : Outport  Port=105
      settings: EnsureOutportIsVirtual=on
  MoleculeVlv2Ngcc_MoleculeVlv2Ngcc_write : Outport  Port=106
      settings: EnsureOutportIsVirtual=on
  MosOvrTempErrNgcc_MosOvrTempErrNgcc_write : Outport  Port=107
      settings: EnsureOutportIsVirtual=on
  MosTempFbNgcc_MosTempFbNgcc_write : Outport  Port=108
      settings: EnsureOutportIsVirtual=on
  MosTempSnsrErrNgcc_MosTempSnsrErrNgcc_write : Outport  Port=109
      settings: EnsureOutportIsVirtual=on
  MotStsFrmOGMNgcc_MotStsFrmOGMNgcc_write : Outport  Port=110
      settings: EnsureOutportIsVirtual=on
  MotTempFbNgcc_MotTempFbNgcc_write : Outport  Port=111
      settings: EnsureOutportIsVirtual=on
  MotTempSnsrErrNgcc_MotTempSnsrErrNgcc_write : Outport  Port=112